simulink model "FluxModel2015a_verify_Q_zhixin"  (Simulink 10.2)
settings: Solver=VariableStepAuto  StartTime=0.0  StopTime=0.5  FixedStep=Ts  RelTol=1e-3  EnableMultiTasking=on
model workspace (MAT data in the model file):
  MOVE = Simulink.Parameter
  MOVE.Value = 1
  MOVE.DataType = 'boolean'
  MOVE.Dimensions = [1 1]
  STOP = Simulink.Parameter
  STOP.Value = 0
  STOP.DataType = 'boolean'
  STOP.Dimensions = [1 1]
  thres = Simulink.Parameter
  thres.Value = 0.1
  thres.DataType = 'double'
  thres.Dimensions = [1 1]
values from neighbouring files (symbols this model uses):
  AngleEncoderEn = 1    [parameters.m]
  AngleSpdClosedLoopEnable = 1.0    (= 1)    [parameters.m]
  CurrentLoopMax = Q14*1.2    (= 19660.8)    [parameters.m]
  EstOutMax_Speed_Q = Q14*1.2    (= 19660.8)    [parameters.m]
  Factor1 = Q15*Ts*Rb/Lq_realvalue    [parameters.m]
  Factor2 = Q15*Ts*Rs_realvalue/Lq_realvalue    (= 655.34)    [parameters.m]
  fieldweaken_Max = 0    [parameters.m]
  fieldweaken_Min = -0.5*Q14    (= -8192)    [parameters.m]
  FieldWeakenEn = 0    [parameters.m]
  fluxF = Lam_realvalue/fluxB    [parameters.m]
  fluxF_Q14 = Q14*fluxF    [parameters.m]
  Friction = 0.003    [parameters.m]
  Ib = 1.5*In    (= 24)    [parameters.m]
  Id_Ki = Q14*CurrentLoopBandwidth*Rs    [parameters.m]
  Id_Kp = Q14*CurrentLoopBandwidth*Ld    [parameters.m]
  IFCurrDecreased = 1    [parameters.m]
  IFCurrStart = 8    [parameters.m]
  IFCurrTarget = 5    [parameters.m]
  IFIdref = 0    [parameters.m]
  initial_degree = 0/Np    (= 0)    [parameters.m]
  Iq_Ki = Q14*CurrentLoopBandwidth*Rs    [parameters.m]
  Iq_Kp = Q14*CurrentLoopBandwidth*Lq    [parameters.m]
  J_realvalue = 0.69e-4    (= 6.9e-05)    [parameters.m]
  KI_fieldweaken = 0.1*Q14    (= 1638.4)    [parameters.m]
  KP_fieldweaken = 1.0*Q14    (= 16384)    [parameters.m]
  LamObserverGain = 0.8    [parameters.m]
  Ld = Ld_realvalue/Lb    [parameters.m]
  Ld_Q14 = Q14*Ld_realvalue/Lb    [parameters.m]
  Ld_realvalue = 0.25e-3    (= 0.00025)    [parameters.m]
  LowPassFilterBandWidth_Emf_Q = Q14*LowPassFilterBandWidth_Emf    (= 4915.2)    [parameters.m]
  LowPassFilterCoeff_Emf = Q15*(1/(1+LowPassFilterBandWidth_Emf*TsPu))    (= 30944.2341498)    [parameters.m]
  LowPassFilterCoeff_Spd = Q15*(1/(1+LowPassFilterBandWidth_Speed*SpeedloopTs/tb))    (= 20123.2126159)    [parameters.m]
  Lq = Lq_realvalue/Lb    [parameters.m]
  Lq_realvalue = 0.25e-3    (= 0.00025)    [parameters.m]
  MOVE = 1    [parameters.m]
  Np = 5    [parameters.m]
  Omega0 = 0.4    [parameters.m]
  OverModulationEn = 0    [parameters.m]
  PLL_KI = Q14*PLL_Bandwidth*PLL_Bandwidth    (= 655.36)    [parameters.m]
  PLL_KP = Q14*2*PLL_Bandwidth    (= 6553.6)    [parameters.m]
  PLL_OUTMax_W = Q14*1.2    (= 19660.8)    [parameters.m]
  PWM_Period = 1000    [parameters.m]
  Q14 = 16384    [parameters.m]
  Q15 = 32767    [parameters.m]
  Rs = Rs_realvalue/Rb    [parameters.m]
  Rs_Q14 = Q14*Rs_realvalue/Rb    [parameters.m]
  Rs_realvalue = 0.08    [parameters.m]
  SMO_Gain = Q14*0.85    (= 13926.4)    [parameters.m]
  SMO_I_ERROR = Q14*0.05    (= 819.2)    [parameters.m]
  SpdClosedLoopEnable = 1.0    (= 1)    [parameters.m]
  Speed_Ki = Q14*SpeedLoopBandwidth    (= 327.68)    [parameters.m]
  Speed_Kp = Q14*SpeedLoopBandwidth*JPu/fluxF    [parameters.m]
  SpeedIncreased = 0.1*Wb    (= 314.159265359)    [parameters.m]
  SpeedloopTs = 1/SpeedloopFrequency    (= 0.001)    [parameters.m]
  SpeedOutmax = 1.2*Q14    (= 19660.8)    [parameters.m]
  SpeedSwitch = 0.6*Wb    (= 1884.95559215)    [parameters.m]
  SpeedTarget = 0.8*Wb    (= 2513.27412287)    [parameters.m]
  STOP = 0    [parameters.m]
  SVCM_GAIN_Q = Q15*SVCM_GAIN    (= 26213.6)    [parameters.m]
  tb = 1/Wb    (= 0.000318309886184)    [parameters.m]
  Tf = 0    [parameters.m]
  thres = 0.1    [parameters.m]
  Ts = 1/SwitchFrequency    (= 6.25e-05)    [parameters.m]
  TsPu = Ts/tb    (= 0.196349540849)    [parameters.m]
  Vb = Un/sqrt(3)    [parameters.m]
  Vdc = 36    [parameters.m]
  Wb = 2*pi*fn    (= 3141.59265359)    [parameters.m]
undefined symbols (not defined in the file or in its folder): DiscIntNeverNeededParam, EstOutMax_Theta, magnitude, sigbuilder_block
libraries: simulink x7, powerlib x5
(part 1 of 2)
settings Model: Creator=Administrator  LastModifiedBy=wangdi  OrderedModelArguments=off
settings DataIOCC: LimitDataPoints=on  SignalLoggingSaveFormat=Dataset
settings OptimizationCC: ExpressionDepthLimit=2147483647  InitFltsAndDblsToZero=on
settings DebuggingCC: SaveWithParameterizedLinksMsg=none  SolverPrmCheckMsg=warning  StateNameClashWarn=warning
settings SFSimCC: SimParseCustomCode=off
settings GRTTargetCC: TargetFcnLib=ansi_tfl_tmw.mat
settings SimscapeCC: SimscapeNormalizeSystem=off

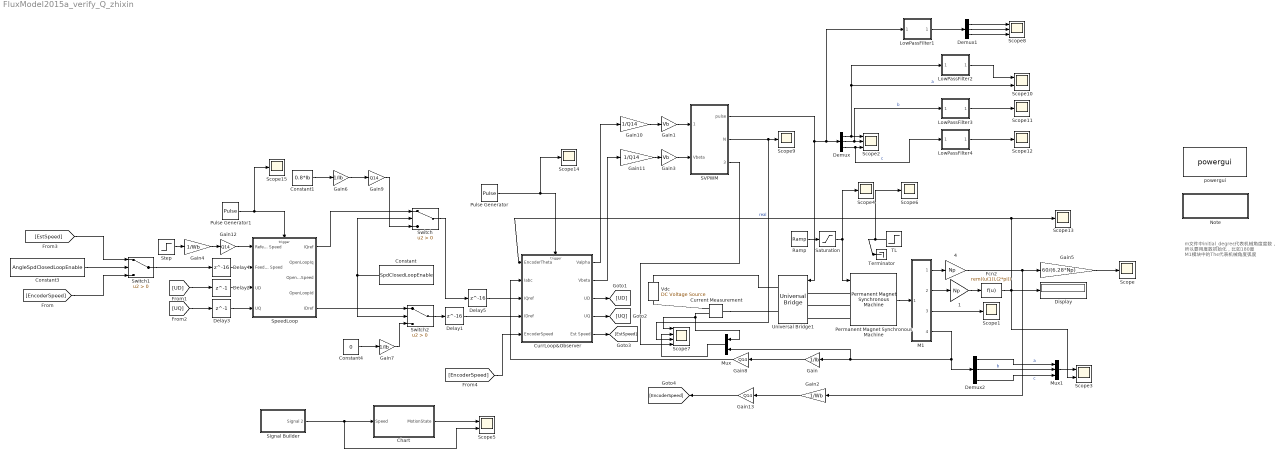
== system FluxModel2015a_verify_Q_zhixin ==
  note: m文件中initial_degree代表机械角度度数，
      | 所以要用度数初始化，比如180度
      | M1模块中的The代表机械角度弧度
  1 : Gain  Gain=Np
  4 : Gain  Gain=Np
  Chart : Stateflow chart  TreatAsAtomicUnit=on
  Constant : Constant  Value=SpdClosedLoopEnable
  Constant1 : Constant  Value=0.8*Ib
  Constant3 : Constant  Value=AngleSpdClosedLoopEnable
  Constant4 : Constant  Value=0
  CurrLoop&Observer : Subsystem
  Current Measurement : powerlib/Measurements/Current Measurement  PhasorSimulation=off  OutputType=Complex  PSBequivalent=0
  Delay1 : Delay  DelayLength=16
  Delay2 : Delay  DelayLength=1
  Delay3 : Delay  DelayLength=1
  Delay4 : Delay  DelayLength=16
  Delay5 : Delay  DelayLength=16
  Demux : Demux  Outputs=3
  Demux1 : Demux  Outputs=3
  Demux2 : Demux  Outputs=3
  Display : Display
  Fcn2 : Fcn  Expr=rem((u(1)),(2*pi))
  Gain : Gain  Gain=1/Ib
  Gain1 : Gain  Gain=Vb
  Gain10 : Gain  Gain=1/Q14
  Gain11 : Gain  Gain=1/Q14
  Gain{8..9,12..13} (4 alike) : Gain  Gain=Q14
  Gain2 : Gain  Gain=1/Wb
  Gain3 : Gain  Gain=Vb
  Gain4 : Gain  Gain=1/Wb
  Gain5 : Gain  Gain=60/(6.28*Np)
  Gain6 : Gain  Gain=1/Ib
  Gain7 : Gain  Gain=1/Ib
  LowPassFilter1 : Subsystem
  LowPassFilter2 : Subsystem
  LowPassFilter3 : Subsystem
  LowPassFilter4 : Subsystem
  M1 : Subsystem
  Mux : Mux  Inputs=2
  Mux1 : Mux  Inputs=3
  Note : Subsystem
  Permanent Magnet Synchronous Machine : powerlib/Machines/Permanent Magnet Synchronous Machine  NbPhases=3  FluxDistribution=Sinusoidal  RotorType=Salient-pole  MechanicalLoad=Torque Tm  PresetModel=No  ShowDetailedParameters=on  MeasurementBus=off  Resistance=Rs_realvalue  Inductance=8.5e-3  dqInductances=[Ld_realvalue, Lq_realvalue]  La=0.000835  MachineConstant=Flux linkage established by magnets (V.s)  Flux=Lam_realvalue  VoltageCst=8.7671  TorqueCst=0.072503  Flat=120  Mechanical=[J_realvalue Friction Np Tf]  PolePairs=Np  InitialConditions=[0,initial_degree, 0,0]  InitialConditions5ph=[0 0 0 0 0 0]  RefAngle=Aligned with phase A axis (original Park)  IterativeDiscreteModel=Trapezoidal non iterative  TsBlock=-1  TsPowergui=0
  Pulse Generator : DiscretePulseGenerator  PulseType=Time based  Period=Ts  PulseWidth=50
  Pulse Generator1 : DiscretePulseGenerator  PulseType=Time based  Period=SpeedloopTs  PulseWidth=50
  Ramp : simulink/Sources/Ramp  slope=20  start=0  InitialOutput=0
  SVPWM : Subsystem
  Saturation : Saturate
  Scope{,1,4,6,9,11..15} (10 alike) : Scope
  Scope10 : Scope  NumInputPorts=2
  Scope2 : Scope  NumInputPorts=3
  Scope3 : Scope  NumInputPorts=2
  Scope5 : Scope  NumInputPorts=2
  Scope7 : Scope  NumInputPorts=4
  Scope8 : Scope  NumInputPorts=3
  Signal Builder : Subsystem  Tag=STV Subsys  LoadFcn=sigbuilder_block('load');  StartFcn=sigbuilder_block('start');  StopFcn=sigbuilder_block('stop');  ClipboardFcn=sigbuilder_block('clipboard');  mask(Sigbuilder block){tuvar=[0:10]}
      mask help: Simulink:masks:SigbuilderBlock_MD
      mask init:
      | if ~strcmp(get_param(bdroot(gcbh),'SimulationStatus'),'stopped') tuvar = sigbuilder_block('maskInit'); end
      settings: CopyFcn=sigbuilder_block('copy');  DeleteFcn=sigbuilder_block('delete');  ModelCloseFcn=sigbuilder_block('modelClose');  PreSaveFcn=sigbuilder_block('preSave');  PauseFcn=sigbuilder_block('pause');  ContinueFcn=sigbuilder_block('continue');  NameChangeFcn=sigbuilder_block('namechange');  OpenFcn=sigbuilder_block('open',[1951.5 -31.5 550.5 326 ]);  CloseFcn=sigbuilder_block('close');
      mask Display: plot(0, 0, 100, 100,[2, 2, 32, 32, 2], [68, 8, 8, 68, 68],[32, 2], [38, 38], [32, 19, 2],[53, 60, 44], [32, 17, 17, 2],[16, 16, 31, 31]);txt = getActiveGroup(gcbh);text(2, 100, txt,'verticalAlignment', 'top');
  SpeedLoop : Subsystem
  Step : Step  Time=0  After=0.5*Wb  SampleTime=0
  Switch : Switch  Criteria=u2 > Threshold
  Switch1 : Switch  Criteria=u2 > Threshold
  Switch2 : Switch  Criteria=u2 > Threshold
  TL : Step  Time=0  Before=1  After=2.88  SampleTime=0
  Terminator : Terminator
  Universal Bridge1 : powerlib/Power Electronics/Universal Bridge  Arms=3  SnubberResistance=1e5  SnubberCapacitance=inf  Device=IGBT / Diodes  Ron=1e-3  Lon=0  ForwardVoltages="[  0  0  ]"  ForwardVoltage=0  GTOparameters=[ 10e-6 , 20e-6 ]  IGBTparameters=[ 1e-6 , 2e-6 ]  Measurements=None  Measurements_2=None  converterType=Rectifier
  Vdc : powerlib/Electrical Sources/DC Voltage Source  Amplitude=Vdc  Measurements=None
  powergui : powerlib/powergui  SimulationMode=Discrete  SampleTime=Ts/100  frequency=60  Iterations=50  frequencyindice=0  Pbase=100e6  ErrMax=1e-4  UnitsV=kV  UnitsW=MW  buscounter=0  FunctionMessages=off  echomessages=off  EnableUseOfTLC=off  CurrentSourceSwitches=off  DisableSnubberDevices=off  DisableRonSwitches=off  DisableVfSwitches=off  DisplayEquations=off  AutomaticDiscreteSolvers=off  SolverType=Tustin/Backward Euler (TBE)  Interpol=off  ExternalGateDelay=off  methode=off  Ts=0  x0status=blocks  SPID=off  SwTol=0  HookPort=off  NonlinearTolerance=1e-4  nMaxIteration=100  ContinueOnMaxIteration=off  RestoreLinks=warning  ResistiveCurrentMeasurement=off  Frange=[0:2:500]  Ylog=off  Xlog=on  ShowGrid=off  save=off  variable=ZData  structure=0  ZoomFFT=off  StartTime=0.0  cycles=1  DisplayStyle=1  fundamental=60  FreqAxis=off  MaxFrequency=1000  frequencyindicesteady=1  RmsSteady=1  display=off
  wires:
  M1/4(Iabc) -> Demux2/1, Mux/2, Gain/1
  M1/2(The) -> 1/1
  Permanent Magnet Synchronous Machine/1 -> M1/1(In1)
  1/1 -> Fcn2/1
  M1/1(wm) -> 4/1
  M1/3(Te) -> Scope1/1
  4/1 -> Gain5/1, Gain2/1
  Fcn2/1 -> Scope3/2, Display/1, CurrLoop&Observer/1(EncoderTheta), Scope13/1  "real"
  Pulse Generator/1 -> Scope14/1, CurrLoop&Observer/Trigger
  SVPWM/1(pulse) -> LowPassFilter1/1(Input), Demux/1, Universal Bridge1/1
  Saturation/1 -> Permanent Magnet Synchronous Machine/1, Scope4/1
  Demux2/1 -> Mux1/1  "a"
  Demux2/2 -> Mux1/2  "b"
  Demux2/3 -> Mux1/3  "c"
  Demux/1 -> Scope10/2, LowPassFilter2/1(Input), Scope2/1  "a"
  Demux/2 -> LowPassFilter3/1(Input), Scope2/2  "b"
  Demux/3 -> LowPassFilter4/1(Input), Scope2/3  "c"
  Ramp/1 -> Saturation/1
  TL/1 -> Scope6/1, Terminator/1
  Gain/1 -> Gain8/1
  CurrLoop&Observer/1(Valpha) -> Gain10/1
  Gain1/1 -> SVPWM/1(Valpha)
  Gain3/1 -> SVPWM/2(Vbeta)
  CurrLoop&Observer/2(Vbeta) -> Gain11/1
  Current Measurement/1 -> Mux/1, Scope7/1
  Mux/1 -> Scope7/2
  SVPWM/2(N) -> Scope7/3, Scope9/1
  SVPWM/3(Output) -> Scope7/4
  LowPassFilter1/1(Output) -> Demux1/1
  Demux1/1 -> Scope8/1
  Demux1/2 -> Scope8/2
  Demux1/3 -> Scope8/3
  LowPassFilter2/1(Output) -> Scope10/1
  LowPassFilter3/1(Output) -> Scope11/1
  LowPassFilter4/1(Output) -> Scope12/1
  Mux1/1 -> Scope3/1
  Gain5/1 -> Scope/1
  Pulse Generator1/1 -> Scope15/1, SpeedLoop/Trigger
  Gain6/1 -> Gain9/1
  Constant1/1 -> Gain6/1
  CurrLoop&Observer/3(UD) -> [UD]
  CurrLoop&Observer/4(UQ) -> [UQ]
  [UD] -> Delay2/1
  [UQ] -> Delay3/1
  SpeedLoop/1(IQref) -> Switch/1
  Step/1 -> Gain4/1
  CurrLoop&Observer/5(Est Speed) -> [EstSpeed]
  Constant3/1 -> Switch1/2
  [EstSpeed] -> Switch1/1
  [EncoderSpeed] -> Switch1/3
  [EncoderSpeed] -> CurrLoop&Observer/5(EncoderSpeed)
  Delay2/1 -> SpeedLoop/3(UD)
  Delay3/1 -> SpeedLoop/4(UQ)
  Delay4/1 -> SpeedLoop/2(Feedback Speed)
  Switch/1 -> Delay5/1
  Switch2/1 -> Delay1/1
  Signal Builder/1(Signal 2) -> Scope5/2, Chart/1(Speed)
  Chart/1(MotionState) -> Scope5/1
  Constant/1 -> Switch2/2, Switch/2
  Constant4/1 -> Gain7/1
  Gain7/1 -> Switch2/3
  Gain8/1 -> CurrLoop&Observer/2(Iabc)
  Gain9/1 -> Switch/3
  Gain10/1 -> Gain1/1
  Gain11/1 -> Gain3/1
  Gain4/1 -> Gain12/1
  Gain2/1 -> Gain13/1
  Delay5/1 -> CurrLoop&Observer/3(IQref)
  Delay1/1 -> CurrLoop&Observer/4(IDref)
  Switch1/1 -> Delay4/1
  Gain12/1 -> SpeedLoop/1(Reference Speed)
  Gain13/1 -> [EncoderSpeed]
  SpeedLoop/5(IDref) -> Switch2/1
  connections:
  Permanent Magnet Synchronous Machine/LConn3 -- Universal Bridge1/LConn3
  Vdc/RConn1 -- Universal Bridge1/RConn1
  Permanent Magnet Synchronous Machine/LConn1 -- Universal Bridge1/LConn1
  Permanent Magnet Synchronous Machine/LConn2 -- Universal Bridge1/LConn2
  Current Measurement/LConn1 -- Universal Bridge1/RConn2
  Current Measurement/RConn1 -- Vdc/LConn1

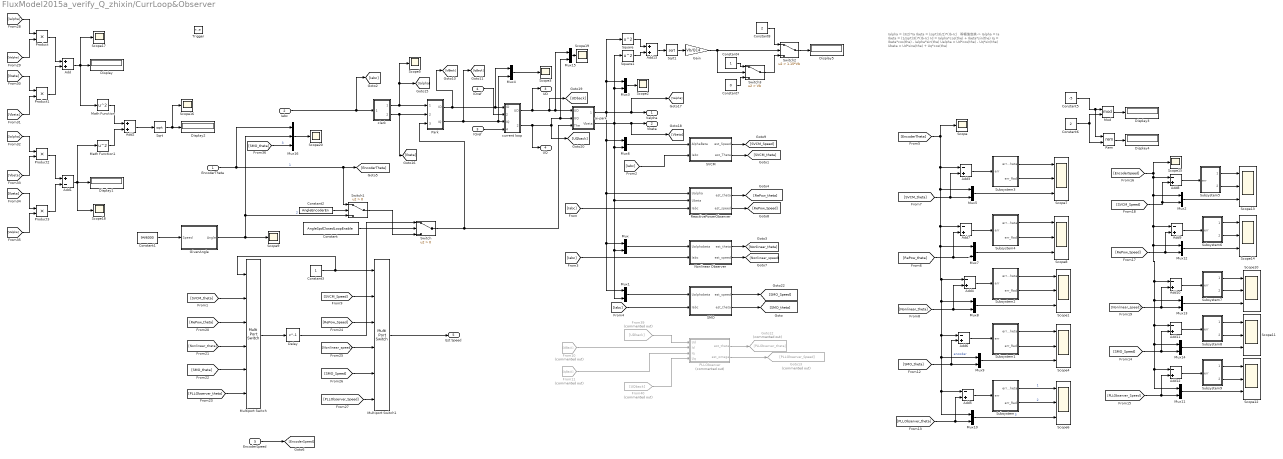
== system FluxModel2015a_verify_Q_zhixin/CurrLoop&Observer ==
  note: Ialpha = (3/2)*Ia
      | Ibeta = (sqrt(3)/2)*(Ib-Ic)
      | 等幅值变换->
      | Ialpha = Ia
      | Ibeta = (1/sqrt(3))*(Ib-Ic)
      |
      | Id = Ialpha*cos(the) + Ibeta*sin(the)
      | Iq = Ibeta*cos(the) - Ialpha*sin(the)
      |
      | Ualpha = Ud*cos(the) - Uq*sin(the)
      | Ubeta = Ud*sine(the) + Uq*cos(the)
  EncoderTheta : Inport
  Iabc : Inport  Port=2
  IQref : Inport  Port=3
  IDref : Inport  Port=4
  EncoderSpeed : Inport  Port=5
  Trigger : TriggerPort  VariantControl=(inherit)
  Add : Sum
  Add1 : Sum  Inputs=+-
  Add{3..12} (10 alike) : Sum  Inputs=-+
  Add13 : Sum
  Add2 : Sum
  Constant : Constant  Value=AngleSpdClosedLoopEnable
  Constant1 : Constant  Value=94/6000
  Constant2 : Constant  Value=AngleEncoderEn
  Constant3 : Constant
  Constant4 : Constant
  Constant5 : Constant  Value=-5
  Constant6 : Constant  Value=2
  Constant7 : Constant  Value=0
  Constant8 : Constant  Value=2
  Delay : Delay  DelayLength=1
  Display{,1..5} (6 alike) : Display
  From10 : From  Commented=on  GotoTag=IdBack
  From11 : From  Commented=on  GotoTag=IqBack
  From39 : From  Commented=on  GotoTag=UDback
  From40 : From  Commented=on  GotoTag=UQback
  Gain : Gain  Gain=Vb/Q14
  GivenAngle : Subsystem
  Goto12 : Goto  Commented=on  GotoTag=PLLObserver_theta
  Goto13 : Goto  Commented=on  GotoTag=PLLObserver_Speed
  Math Function : Math  Operator=square  SaturateOnIntegerOverflow=on
  Math Function1 : Math  Operator=square  SaturateOnIntegerOverflow=on
  Mod : Math  Operator=mod  SaturateOnIntegerOverflow=on
  Multiport Switch : MultiPortSwitch  Inputs=5
  Multiport Switch1 : MultiPortSwitch  Inputs=5
  Mux{,1..15} (16 alike) : Mux  Inputs=2
  Mux16 : Mux  Inputs=3
  Nonlinear Observer : Subsystem
  PLLObserver : Subsystem  Commented=on
  Park : Subsystem
  Product{,1..3} (4 alike) : Product
  ReactivePowerObserver : Subsystem
  Rem : Math  Operator=rem  SaturateOnIntegerOverflow=on
  SMO : Subsystem
  SVCM : Subsystem
  Scope{,2..3,5,9,15..20} (11 alike) : Scope
  Scope{1,4,6..8,10..14} (10 alike) : Scope  NumInputPorts=3
  Sqrt : Sqrt  SaturateOnIntegerOverflow=on
  Sqrt1 : Sqrt  SaturateOnIntegerOverflow=on
  Square : Math  Operator=square  SaturateOnIntegerOverflow=on
  Square1 : Math  Operator=square  SaturateOnIntegerOverflow=on
  Subsystem : Subsystem
  Subsystem1 : Subsystem
  Subsystem2 : Subsystem
  Subsystem3 : Subsystem
  Subsystem4 : Subsystem
  Subsystem5 : Subsystem
  Subsystem6 : Subsystem
  Subsystem7 : Subsystem
  Subsystem8 : Subsystem
  Subsystem9 : Subsystem
  Switch : Switch  Criteria=u2 > Threshold
  Switch1 : Switch  Criteria=u2 > Threshold
  Switch2 : Switch  Criteria=u2 > Threshold  Threshold=1.15*Vb
  Switch3 : Switch  Criteria=u2 > Threshold  Threshold=Vb
  ai-park : Subsystem
  clark : Subsystem
  current loop : Subsystem
  Valpha : Outport
  Vbeta : Outport  Port=2
  UD : Outport  Port=3
  UQ : Outport  Port=4
  Est Speed : Outport  Port=5
  wires:
  Iabc/1 -> [Iabc], clark/1(Iabc)
  Park/1(ID) -> Mux4/1, current loop/1(ID), [IdBack]
  Park/2(IQ) -> Mux4/2, current loop/3(IQ), [IqBack]
  Mux3/1 -> Scope2/1
  EncoderTheta/1 -> Mux16/1, Switch1/1, [EncoderTheta]  "1"
  ai-park/1(Valpha) -> ReactivePowerObserver/1(Ualpha), Mux1/1, Mux/1, Mux6/1, [Valpha], Valpha/1, Square/1, Mux3/1
  ai-park/2(Vbeta) -> ReactivePowerObserver/2(Ubeta), Mux1/2, Mux/2, Mux6/2, [Vbeta], Vbeta/1, Square1/1, Mux3/2
  [Iabc] -> ReactivePowerObserver/3(Iabc)
  Mux/1 -> Nonlinear Observer/1(Ualphabeta)
  [Iabc] -> Nonlinear Observer/2(Iabc)
  Nonlinear Observer/1(est_theta) -> [Nonlinear_theta]
  ReactivePowerObserver/1(est_theta) -> [RePow_theta]
  Mux1/1 -> SMO/1(Ualphabeta)
  [Iabc] -> SMO/2(Iabc)
  Mux7/1 -> Scope8/3
  Nonlinear Observer/2(est_speed) -> [Nonlinear_speed]
  ReactivePowerObserver/2(est_speed) -> [RePow_Speed]
  clark/1(Ialpha) -> Scope5/1, [Ialpha], Park/1(alpha)
  clark/2(Ibeta) -> [Ibeta], Park/2(beta)
  From10/1 -> PLLObserver/2(Id)
  From11/1 -> PLLObserver/3(Iq)
  [PLLObserver_theta] -> Add5/2, Mux10/2
  [EncoderSpeed] -> Scope15/1, Add8/1, Mux2/1, Add10/1, Mux13/1, Add11/1, Mux14/1, Mux11/1, Add12/1, Mux12/1, Add9/1
  [SVCM_theta] -> Multiport Switch/2
  [RePow_theta] -> Multiport Switch/3
  [Nonlinear_theta] -> Multiport Switch/4
  [SMO_theta] -> Multiport Switch/5
  [PLLObserver_theta] -> Multiport Switch/6
  Constant3/1 -> Multiport Switch/1, Multiport Switch1/1
  [SVCM_Speed] -> Multiport Switch1/2
  [RePow_Speed] -> Multiport Switch1/3
  [Nonlinear_speed] -> Multiport Switch1/4
  [SMO_Speed] -> Multiport Switch1/5
  [PLLObserver_Speed] -> Multiport Switch1/6
  [Ialpha] -> Product/1
  [Valpha] -> Product/2
  [Vbeta] -> Product1/2
  [Ibeta] -> Product1/1
  Product/1 -> Add/1
  Product1/1 -> Add/2
  Add/1 -> Scope17/1, Math Function/1, Display/1
  Add1/1 -> Scope18/1, Math Function1/1, Display1/1
  Product3/1 -> Add1/2
  Product2/1 -> Add1/1
  [Ibeta] -> Product3/1
  [Valpha] -> Product3/2
  [Vbeta] -> Product2/2
  [Ialpha] -> Product2/1
  Math Function/1 -> Add2/1
  Math Function1/1 -> Add2/2
  Add2/1 -> Sqrt/1
  Sqrt/1 -> Scope16/1, Display2/1
  IDref/1 -> current loop/2(IDref)
  IQref/1 -> current loop/4(IQref)
  [SMO_theta] -> Mux9/2, Add6/2
  [EncoderTheta] -> Add3/1, Mux5/1, Add7/1, Mux8/1, Add6/1, Mux9/1, Add5/1, Mux10/1, Add4/1, Mux7/1, Scope/1  "encoder"
  SMO/1(est_speed) -> [SMO_Speed]
  SMO/2(est_theta) -> [SMO_theta]
  Constant5/1 -> Rem/1, Mod/1
  Constant6/1 -> Mod/2, Rem/2
  Mod/1 -> Display3/1
  Rem/1 -> Display4/1
  Add5/1 -> Subsystem/1(err)
  Subsystem/1(err_Theta) -> Scope6/1  "1"
  Subsystem/2(err_Rad) -> Scope6/2  "2"
  Mux10/1 -> Scope6/3  "3"
  Add6/1 -> Subsystem1/1(err)
  Subsystem1/1(err_Theta) -> Scope4/1
  Subsystem1/2(err_Rad) -> Scope4/2
  Add4/1 -> Subsystem2/1(err)
  Subsystem2/1(err_Theta) -> Scope1/1
  Subsystem2/2(err_Rad) -> Scope1/2
  Mux8/1 -> Scope1/3
  [Nonlinear_theta] -> Add4/2, Mux8/2
  Add3/1 -> Subsystem3/1(err)
  Subsystem3/1(err_Theta) -> Scope7/1
  Subsystem3/2(err_Rad) -> Scope7/2
  Mux5/1 -> Scope7/3
  [SVCM_theta] -> Add3/2, Mux5/2
  Add7/1 -> Subsystem4/1(err)
  Subsystem4/1(err_Theta) -> Scope8/1
  Subsystem4/2(err_Rad) -> Scope8/2
  [RePow_theta] -> Add7/2, Mux7/2
  Add8/1 -> Subsystem5/1(err)
  Subsystem5/1(err_Spd) -> Scope13/1
  Subsystem5/2(err_Fhz) -> Scope13/2
  Mux2/1 -> Scope13/3
  [SVCM_Speed] -> Add8/2, Mux2/2
  Add9/1 -> Subsystem6/1(err)
  Subsystem6/1(err_Spd) -> Scope14/1
  Subsystem6/2(err_Fhz) -> Scope14/2
  Mux12/1 -> Scope14/3
  [RePow_Speed] -> Add9/2, Mux12/2
  Add10/1 -> Subsystem7/1(err)
  Subsystem7/1(err_Spd) -> Scope10/1
  Subsystem7/2(err_Fhz) -> Scope10/2
  Mux13/1 -> Scope10/3
  [Nonlinear_speed] -> Add10/2, Mux13/2
  Add11/1 -> Subsystem8/1(err)
  Subsystem8/1(err_Spd) -> Scope11/1
  Subsystem8/2(err_Fhz) -> Scope11/2
  Mux14/1 -> Scope11/3
  [SMO_Speed] -> Add11/2, Mux14/2
  Add12/1 -> Subsystem9/1(err)
  Subsystem9/1(err_Spd) -> Scope12/1
  Subsystem9/2(err_Fhz) -> Scope12/2
  Mux11/1 -> Scope12/3
  [PLLObserver_Speed] -> Add12/2, Mux11/2
  PLLObserver/1(est_theta) -> Goto12/1
  PLLObserver/2(est_omega) -> Goto13/1
  current loop/1(UD) -> [UDback], Mux15/1, ai-park/1(UD), UD/1
  current loop/2(UQ) -> UQ/1, Mux15/2, ai-park/2(UQ), [UQback]
  From39/1 -> PLLObserver/1(Ud)
  From40/1 -> PLLObserver/4(Uq)
  Multiport Switch1/1 -> Est Speed/1
  Constant/1 -> Switch/2
  EncoderSpeed/1 -> [EncoderSpeed]
  Constant1/1 -> GivenAngle/1(Speed)
  GivenAngle/1(Angle) -> Scope9/1, Switch1/3, Mux16/2  "2"
  Mux4/1 -> Scope3/1
  Mux15/1 -> Scope19/1
  Mux9/1 -> Scope4/3
  [SMO_theta] -> Mux16/3  "3"
  Mux16/1 -> Scope20/1
  Constant2/1 -> Switch1/2
  Switch1/1 -> Switch/3
  Multiport Switch/1 -> Delay/1
  Switch/1 -> Park/3(theta), ai-park/3(The)
  [Iabc] -> SVCM/2(Iabc)
  Mux6/1 -> SVCM/1(AlphaBeta)
  SVCM/1(est_Speed) -> [SVCM_Speed]
  SVCM/2(est_Theta) -> [SVCM_theta]
  Square/1 -> Add13/1
  Square1/1 -> Add13/2
  Add13/1 -> Sqrt1/1
  Sqrt1/1 -> Gain/1
  Switch2/1 -> Display5/1
  Constant8/1 -> Switch2/1
  Switch3/1 -> Switch2/3
  Constant4/1 -> Switch3/1
  Constant7/1 -> Switch3/3
  Gain/1 -> Switch3/2, Switch2/2
  Delay/1 -> Switch/1

== system FluxModel2015a_verify_Q_zhixin/LowPassFilter1 ==
  Input : Inport
  Add : Sum  Inputs=+-
  Add1 : Sum
  Constant : Constant
  Constant1 : Constant  Value=1/(1+20*Ts)
  Delay6 : Delay  DelayLength=1
  Gain : Gain  Gain=1/(1+20*Ts)
  Product : Product
  Output : Outport
  wires:
  Add1/1 -> Output/1, Delay6/1
  Gain/1 -> Add1/2
  Product/1 -> Add1/1
  Add/1 -> Product/2
  Input/1 -> Product/1
  Constant1/1 -> Add/2
  Constant/1 -> Add/1
  Delay6/1 -> Gain/1

== system FluxModel2015a_verify_Q_zhixin/LowPassFilter2 ==
  Input : Inport
  Add : Sum  Inputs=+-
  Add1 : Sum
  Constant : Constant
  Constant1 : Constant  Value=1/(1+20*Ts)
  Delay6 : Delay  DelayLength=1
  Gain : Gain  Gain=1/(1+20*Ts)
  Product : Product
  Output : Outport
  wires:
  Add1/1 -> Output/1, Delay6/1
  Gain/1 -> Add1/2
  Product/1 -> Add1/1
  Add/1 -> Product/2
  Input/1 -> Product/1
  Constant1/1 -> Add/2
  Constant/1 -> Add/1
  Delay6/1 -> Gain/1

== system FluxModel2015a_verify_Q_zhixin/LowPassFilter3 ==
  Input : Inport
  Add : Sum  Inputs=+-
  Add1 : Sum
  Constant : Constant
  Constant1 : Constant  Value=1/(1+20*Ts)
  Delay6 : Delay  DelayLength=1
  Gain : Gain  Gain=1/(1+20*Ts)
  Product : Product
  Output : Outport
  wires:
  Delay6/1 -> Gain/1
  Constant/1 -> Add/1
  Constant1/1 -> Add/2
  Input/1 -> Product/1
  Add/1 -> Product/2
  Product/1 -> Add1/1
  Gain/1 -> Add1/2
  Add1/1 -> Delay6/1, Output/1

== system FluxModel2015a_verify_Q_zhixin/LowPassFilter4 ==
  Input : Inport
  Add : Sum  Inputs=+-
  Add1 : Sum
  Constant : Constant
  Constant1 : Constant  Value=1/(1+20*Ts)
  Delay6 : Delay  DelayLength=1
  Gain : Gain  Gain=1/(1+20*Ts)
  Product : Product
  Output : Outport
  wires:
  Add1/1 -> Output/1, Delay6/1
  Gain/1 -> Add1/2
  Product/1 -> Add1/1
  Add/1 -> Product/2
  Input/1 -> Product/1
  Constant1/1 -> Add/2
  Constant/1 -> Add/1
  Delay6/1 -> Gain/1

== system FluxModel2015a_verify_Q_zhixin/M1 ==
  In1 : Inport
  Bus Selector : BusSelector  OutputSignals=Stator current is_a (A),Stator current is_b (A),Stator current is_c (A)
  Bus Selector1 : BusSelector  OutputSignals=Rotor speed wm (rad/s),Rotor angle thetam (rad),Electromagnetic torque Te (N*m)
  Mux : Mux  Inputs=3
  wm : Outport
  The : Outport  Port=2
  Te : Outport  Port=3
  Iabc : Outport  Port=4
  wires:
  Bus Selector/1 -> Mux/1  "<Stator current is_a (A)>"
  Bus Selector/2 -> Mux/2  "<Stator current is_b (A)>"
  Bus Selector/3 -> Mux/3  "<Stator current is_c (A)>"
  Bus Selector1/1 -> wm/1  "<Rotor speed wm (rad/s)>"
  Bus Selector1/2 -> The/1  "<Rotor angle thetam (rad)>"
  Bus Selector1/3 -> Te/1  "<Electromagnetic torque Te (N*m)>"
  Mux/1 -> Iabc/1
  In1/1 -> Bus Selector1/1, Bus Selector/1

== system FluxModel2015a_verify_Q_zhixin/Note ==
  note: 多输入开关
  note: 双输入开关
  note: $1.定子三相电压方程：\\ u_a = R_s i_a + \frac{d\psi_a}{dt}\\ u_b = R_s i_b + \frac{d\psi_b}{dt}\\ u_c = R_s i_c + \frac{d\psi_c}{dt}$
  note: 3. 常用模块
  note: 单位延时：Unit Delay
  note: 2.刹车方式：
      | 	a. 短路刹车，若参与PWM抬升母线电压
      | 	b. Iq负电流刹车，抬升母线电压
      | 	c. Id正电流刹车，电机本体发热
  note: 1.30°以内Theta_rads近似Sin(Theta_rads)
  note: 单位延时：Repeating Sequence
  note: 斜坡函数：Ramp
  note: 限幅函数：Saturation
  note: 条件函数：Switch
  note: 积分函数：Discrete-Time Integrator
  note: 功能函数：MATLAB Function
  note: $2.两相静止坐标系电机方程\\ u_\alpha = R_s i_\alpha + L_\alpha\frac{di_\alpha}{dt}+L_{\alpha\beta}\frac{di_\beta}{dt}-\omega_e \psi_rsin(\theta)\\ u_\beta = R_s i_\beta + L_\beta\frac{di_\beta}{dt}+L_{\alpha\beta}\frac{di_\alpha}{dt}+\omega_e \psi_rsin(\theta)\\ L=\frac{1}{2}(L_d+L_q)\\ {\Delta}L=\frac{1}{2}(L_d-L_q)\\ L_\alpha = L + {\Delta}Lcos(2\theta)\\ L_\beta = L - {\Delta}Lcos(2\theta)\\ L_{\alpha\beta}= {\Delta}Lsin(2\theta)\\ \\ 有功功率：P=u_\alpha i_\alpha + u_\beta i_\beta\\ 无功功率：Q=u_\beta i_\alpha - u_\alpha i_\beta\\ 视在功率：S=\sqrt{P^2+Q^2}\\$
  note: $3.旋转坐标系电机方程\\ \psi_d =L_di_d+\psi_r\\ \psi_q=L_qi_q\\ u_d=R_si_d+\frac{d\psi_d}{dt}-\omega_e\psi_q\\ u_q=R_si_q+\frac{d\psi_q}{dt}+\omega_e\psi_d\\ u_d = R_s i_d + L_d\frac{di_d}{dt}-\omega_eL_qi_q\\ u_q = R_s i_q + L_q\frac{di_q}{dt}+\omega_eL_di_d+\omega_e\psi_r\\ \\ 有功功率：P=u_d i_d + u_q i_q\\ 无功功率：Q=u_q i_d - u_d i_q\\ 视在功率：S=\sqrt{P^2+Q^2}\\ 电磁转矩：T_e=\frac{3}{2}P_ni_q[i_d(L_d-L_q)+\psi_r]\\ SPM电磁转矩:T_e=\frac{3}{2}P_n\psi_ri_q$
  note: $运动方程：T_e-T_L=\frac{J}{N_p}\frac{d\omega}{dt}\\ 功率方程：P_t=T_e\omega_r\\ 功率因素：cos(\phi)=\frac{P}{Q}\\ 效率：\eta=\frac{P_t}{P}$
  Discrete-Time Integrator1 : DiscreteIntegrator  Commented=on  gainval=Ts  SampleTime=-1  ICPrevOutput=DiscIntNeverNeededParam  ICPrevScaledInput=DiscIntNeverNeededParam
  MATLAB Function : MATLAB Function  Commented=on  TreatAsAtomicUnit=on
  Manual Switch : ManualSwitch  Commented=on
  Multiport Switch : MultiPortSwitch  Commented=on
  Ramp : simulink/Sources/Ramp  Commented=on  slope=10  start=0  InitialOutput=0
  Ramp1 : simulink/Sources/Ramp  Commented=on  slope=1  start=0  InitialOutput=0
  Repeating Sequence : simulink/Sources/Repeating Sequence  Commented=on  rep_seq_t=[0 2]  rep_seq_y=[0 2]
  Saturation : Saturate  Commented=on  UpperLimit=pi/2  LowerLimit=-pi/2
  Saturation1 : Saturate  Commented=on  UpperLimit=1000  LowerLimit=0
  Scope10 : Scope  Commented=on  NumInputPorts=2
  Sin : Trigonometry  Commented=on
  Switch : Switch  Commented=on  Criteria=u2 > Threshold
  Unit Delay : UnitDelay  Commented=on  SampleTime=-1  HasFrameUpgradeWarning=on
  wires:
  Ramp/1 -> Saturation/1
  Sin/1 -> Scope10/1
  Saturation/1 -> Scope10/2, Sin/1
